simulink model "TosAeb"  (R2021a)
settings: Solver=FixedStepDiscrete  StartTime=0.0  StopTime=inf  FixedStep=0.01  RelTol=1e-3
undefined symbols (not defined in the file or in its folder): ACCModSts, AccSts1, AEB_ObjectMovingOrientation, AEB_ObjectMovingState, CN_BTCActive, CN_CurrentGearR, CN_CurrentGearR_DHT, CN_PTCActive, CN_VDCActive, CN_VSC_EspOff, CN_VSC_FCW_AEB_Error, CN_VSC_FCW_AEB_NoDispay, CN_VSC_FCW_AEB_PerfmDgdtn, CN_VSC_FCW_AEB_TempUnAvlbl, CN_VSC_VehStandstill, CNaccelAvoidMax, CNaccelAvoidMin, CNbrakePedalPress, CNcomfortStrLeftAccelMax, CNcomfortStrLeftAccelMin, CNcomfortStrRightAccelMax, CNcomfortStrRightAccelMin, CNDrvDoorOpen, CNDrvModeEco, CNDrvModeNorm, CNDrvModeOther, CNDrvModeSport, CNDrvSBRreminder, CNNull, CNobjInvalidID, CNPassDoorOpen, CNPassSBRreminder, CNPEBSAccelNegInf, CNPEBSAccelNull, CNPEBSTimeInf, CNPEBSTimeNull, CNSTOPMAX, CNSTOPNULL, CNsubjWidth, CNSwtOFF, CNSwtON, CNTTCMAX, CNTTCNULL, CNVehConfigAEBCycNone, CNVehConfigAEBNone, CNVehConfigAEBPedNone, CNVehConfigFCWNone, CNVehConfigJANone, CollisionSeverity, FALSE, FCWSnvty, JACycObjCalcInfoDefaultParams, JAPedObjCalcInfoDefaultParams, JAVehObjCalcInfoDefaultParams, latCycObjCalcInfoDefaultParams, latPedObjCalcInfoDefaultParams, longObjCalcInfoDefaultParams, ModSts, movingStatus, ObjectType ... (+1112)
libraries: simulink x3031, AEBSFunctionLib x50, Ta_Library x33, AEBSStateMachineLib x23, Mylibrary x12, simulink_extras x7, ObjSelect_lib x3, dspstat3 x2
(part 50 of 102)

== system TosAeb/FcwAebJa_Step_sys/ALG_AEBSystem/ALG_AEBInput/JaObjInfoMap/JaObjInfoMap/Subsystem1/ObjType_Subsystem1/InputObjectType ==
  note: 输出数值为2，类型为car
  note: 输出数值为3，类型为truck
  note: 输出数值为6，类型为pedestrian行人
  note: 输出数值为4，类型为motorbike摩托车
  note: 输出数值为5，类型为bicycle自行车
  note: 输出数值为7，类型为general_object
  note: 输出数值为1，类型为unknown
  InputObjType : Inport
  Compare To Constant : simulink/Logic and Bit Operations/Compare To Constant  relop===  const=0  OutDataTypeStr=boolean
  Compare To Constant1 : simulink/Logic and Bit Operations/Compare To Constant  relop===  const=1  OutDataTypeStr=boolean
  Compare To Constant10 : simulink/Logic and Bit Operations/Compare To Constant  relop===  const=7  OutDataTypeStr=boolean
  Compare To Constant11 : simulink/Logic and Bit Operations/Compare To Constant  relop===  const=9  OutDataTypeStr=boolean
  Compare To Constant12 : simulink/Logic and Bit Operations/Compare To Constant  relop===  const=8  OutDataTypeStr=boolean
  Compare To Constant13 : simulink/Logic and Bit Operations/Compare To Constant  relop===  const=15  OutDataTypeStr=boolean
  Compare To Constant14 : simulink/Logic and Bit Operations/Compare To Constant  relop===  const=10  OutDataTypeStr=boolean
  Compare To Constant15 : simulink/Logic and Bit Operations/Compare To Constant  relop===  const=11  OutDataTypeStr=boolean
  Compare To Constant16 : simulink/Logic and Bit Operations/Compare To Constant  relop===  const=12  OutDataTypeStr=boolean
  Compare To Constant17 : simulink/Logic and Bit Operations/Compare To Constant  relop===  const=14  OutDataTypeStr=boolean
  Compare To Constant18 : simulink/Logic and Bit Operations/Compare To Constant  relop===  const=12  OutDataTypeStr=boolean
  Compare To Constant2 : simulink/Logic and Bit Operations/Compare To Constant  relop===  const=16  OutDataTypeStr=boolean
  Compare To Constant3 : simulink/Logic and Bit Operations/Compare To Constant  relop===  const=2  OutDataTypeStr=boolean
  Compare To Constant4 : simulink/Logic and Bit Operations/Compare To Constant  relop===  const=3  OutDataTypeStr=boolean
  Compare To Constant5 : simulink/Logic and Bit Operations/Compare To Constant  relop===  const=4  OutDataTypeStr=boolean
  Compare To Constant6 : simulink/Logic and Bit Operations/Compare To Constant  relop===  const=5  OutDataTypeStr=boolean
  Compare To Constant7 : simulink/Logic and Bit Operations/Compare To Constant  relop===  const=18  OutDataTypeStr=boolean
  Compare To Constant8 : simulink/Logic and Bit Operations/Compare To Constant  relop===  const=17  OutDataTypeStr=boolean
  Compare To Constant9 : simulink/Logic and Bit Operations/Compare To Constant  relop===  const=6  OutDataTypeStr=boolean
  Logical Operator : Logic  Operator=OR  Inputs=4  AllPortsSameDT=off  OutDataTypeStr=boolean
  Logical Operator1 : Logic  Operator=OR  Inputs=5  AllPortsSameDT=off  OutDataTypeStr=boolean
  Logical Operator2 : Logic  Operator=OR  AllPortsSameDT=off  OutDataTypeStr=boolean
  Logical Operator3 : Logic  Operator=OR  AllPortsSameDT=off  OutDataTypeStr=boolean
  Logical Operator5 : Logic  Operator=OR  Inputs=4  AllPortsSameDT=off  OutDataTypeStr=boolean
  Input_ObjCar : Outport
  Input_ObjTruck : Outport  Port=2
  Input_ObjPed : Outport  Port=3
  Input_ObjMotor : Outport  Port=4
  Input_ObjBicycle : Outport  Port=5
  Input_ObjGeneral : Outport  Port=6
  Input_Unknown : Outport  Port=7
  wires:
  Compare To Constant12/1 -> Logical Operator3/1
  Compare To Constant6/1 -> Logical Operator1/4
  Logical Operator/1 -> Input_ObjCar/1
  Compare To Constant13/1 -> Logical Operator3/2
  InputObjType/1 -> Compare To Constant8/1, Compare To Constant18/1, Compare To Constant17/1, Compare To Constant16/1, Compare To Constant15/1, Compare To Constant14/1, Compare To Constant13/1, Compare To Constant12/1, Compare To Constant11/1, Compare To Constant10/1, Compare To Constant9/1, Compare To Constant7/1, Compare To Constant6/1, Compare To Constant5/1, Compare To Constant4/1, Compare To Constant3/1, Compare To Constant2/1, Compare To Constant1/1, Compare To Constant/1
  Compare To Constant2/1 -> Logical Operator/3
  Compare To Constant1/1 -> Logical Operator/2
  Compare To Constant16/1 -> Logical Operator5/3
  Compare To Constant18/1 -> Input_Unknown/1
  Compare To Constant15/1 -> Logical Operator5/2
  Compare To Constant10/1 -> Logical Operator2/1
  Logical Operator5/1 -> Input_ObjGeneral/1
  Compare To Constant5/1 -> Logical Operator1/3
  Logical Operator3/1 -> Input_ObjBicycle/1
  Logical Operator1/1 -> Input_ObjTruck/1
  Compare To Constant14/1 -> Logical Operator5/1
  Compare To Constant3/1 -> Logical Operator1/1
  Logical Operator2/1 -> Input_ObjMotor/1
  Compare To Constant7/1 -> Logical Operator1/5
  Compare To Constant4/1 -> Logical Operator1/2
  Compare To Constant11/1 -> Logical Operator2/2
  Compare To Constant8/1 -> Logical Operator/4
  Compare To Constant/1 -> Logical Operator/1
  Compare To Constant17/1 -> Logical Operator5/4
  Compare To Constant9/1 -> Input_ObjPed/1

== system TosAeb/FcwAebJa_Step_sys/ALG_AEBSystem/ALG_AEBInput/LatObjInfoMap/latObjInfoMap/Subsystem/MovingStatus_Subsystem/InputMovingStatus ==
  note: 输出数值为3，类型为Moving
  note: 输出数值为2，类型为Stopped
  note: 输出数值为1，类型为Stationary
  note: 输出数值为0，类型为Unknown
  MovingStatus : Inport
  Compare To Constant : simulink/Logic and Bit Operations/Compare To Constant  relop===  const=0  OutDataTypeStr=boolean
  Compare To Constant1 : simulink/Logic and Bit Operations/Compare To Constant  relop===  const=1  OutDataTypeStr=boolean
  Compare To Constant2 : simulink/Logic and Bit Operations/Compare To Constant  relop===  const=3  OutDataTypeStr=boolean
  Compare To Constant8 : simulink/Logic and Bit Operations/Compare To Constant  relop===  const=2  OutDataTypeStr=boolean
  Input_Unknown : Outport
  Input_Stationary : Outport  Port=2
  Input_Stopped : Outport  Port=3
  Input_Moving : Outport  Port=4
  wires:
  Compare To Constant8/1 -> Input_Moving/1
  Compare To Constant1/1 -> Input_Stationary/1
  Compare To Constant2/1 -> Input_Stopped/1
  MovingStatus/1 -> Compare To Constant/1, Compare To Constant1/1, Compare To Constant2/1, Compare To Constant8/1
  Compare To Constant/1 -> Input_Unknown/1

== system TosAeb/FcwAebJa_Step_sys/ALG_AEBSystem/ALG_AEBInput/LatObjInfoMap/latObjInfoMap/Subsystem/ObjType_Subsystem1/InputObjectType ==
  note: 输出数值为2，类型为car
  note: 输出数值为3，类型为truck
  note: 输出数值为6，类型为pedestrian行人
  note: 输出数值为4，类型为motorbike摩托车
  note: 输出数值为5，类型为bicycle自行车
  note: 输出数值为7，类型为general_object
  note: 输出数值为1，类型为unknown
  InputObjType : Inport
  Compare To Constant : simulink/Logic and Bit Operations/Compare To Constant  relop===  const=0  OutDataTypeStr=boolean
  Compare To Constant1 : simulink/Logic and Bit Operations/Compare To Constant  relop===  const=1  OutDataTypeStr=boolean
  Compare To Constant10 : simulink/Logic and Bit Operations/Compare To Constant  relop===  const=7  OutDataTypeStr=boolean
  Compare To Constant11 : simulink/Logic and Bit Operations/Compare To Constant  relop===  const=9  OutDataTypeStr=boolean
  Compare To Constant12 : simulink/Logic and Bit Operations/Compare To Constant  relop===  const=8  OutDataTypeStr=boolean
  Compare To Constant13 : simulink/Logic and Bit Operations/Compare To Constant  relop===  const=15  OutDataTypeStr=boolean
  Compare To Constant14 : simulink/Logic and Bit Operations/Compare To Constant  relop===  const=10  OutDataTypeStr=boolean
  Compare To Constant15 : simulink/Logic and Bit Operations/Compare To Constant  relop===  const=11  OutDataTypeStr=boolean
  Compare To Constant16 : simulink/Logic and Bit Operations/Compare To Constant  relop===  const=12  OutDataTypeStr=boolean
  Compare To Constant17 : simulink/Logic and Bit Operations/Compare To Constant  relop===  const=14  OutDataTypeStr=boolean
  Compare To Constant18 : simulink/Logic and Bit Operations/Compare To Constant  relop===  const=12  OutDataTypeStr=boolean
  Compare To Constant2 : simulink/Logic and Bit Operations/Compare To Constant  relop===  const=16  OutDataTypeStr=boolean
  Compare To Constant3 : simulink/Logic and Bit Operations/Compare To Constant  relop===  const=2  OutDataTypeStr=boolean
  Compare To Constant4 : simulink/Logic and Bit Operations/Compare To Constant  relop===  const=3  OutDataTypeStr=boolean
  Compare To Constant5 : simulink/Logic and Bit Operations/Compare To Constant  relop===  const=4  OutDataTypeStr=boolean
  Compare To Constant6 : simulink/Logic and Bit Operations/Compare To Constant  relop===  const=5  OutDataTypeStr=boolean
  Compare To Constant7 : simulink/Logic and Bit Operations/Compare To Constant  relop===  const=18  OutDataTypeStr=boolean
  Compare To Constant8 : simulink/Logic and Bit Operations/Compare To Constant  relop===  const=17  OutDataTypeStr=boolean
  Compare To Constant9 : simulink/Logic and Bit Operations/Compare To Constant  relop===  const=6  OutDataTypeStr=boolean
  Logical Operator : Logic  Operator=OR  Inputs=4  AllPortsSameDT=off  OutDataTypeStr=boolean
  Logical Operator1 : Logic  Operator=OR  Inputs=5  AllPortsSameDT=off  OutDataTypeStr=boolean
  Logical Operator2 : Logic  Operator=OR  AllPortsSameDT=off  OutDataTypeStr=boolean
  Logical Operator3 : Logic  Operator=OR  AllPortsSameDT=off  OutDataTypeStr=boolean
  Logical Operator5 : Logic  Operator=OR  Inputs=4  AllPortsSameDT=off  OutDataTypeStr=boolean
  Input_ObjCar : Outport
  Input_ObjTruck : Outport  Port=2
  Input_ObjPed : Outport  Port=3
  Input_ObjMotor : Outport  Port=4
  Input_ObjBicycle : Outport  Port=5
  Input_ObjGeneral : Outport  Port=6
  Input_Unknown : Outport  Port=7
  wires:
  Compare To Constant12/1 -> Logical Operator3/1
  Compare To Constant6/1 -> Logical Operator1/4
  Logical Operator/1 -> Input_ObjCar/1
  Compare To Constant13/1 -> Logical Operator3/2
  InputObjType/1 -> Compare To Constant8/1, Compare To Constant18/1, Compare To Constant17/1, Compare To Constant16/1, Compare To Constant15/1, Compare To Constant14/1, Compare To Constant13/1, Compare To Constant12/1, Compare To Constant11/1, Compare To Constant10/1, Compare To Constant9/1, Compare To Constant7/1, Compare To Constant6/1, Compare To Constant5/1, Compare To Constant4/1, Compare To Constant3/1, Compare To Constant2/1, Compare To Constant1/1, Compare To Constant/1
  Compare To Constant2/1 -> Logical Operator/3
  Compare To Constant1/1 -> Logical Operator/2
  Compare To Constant16/1 -> Logical Operator5/3
  Compare To Constant18/1 -> Input_Unknown/1
  Compare To Constant15/1 -> Logical Operator5/2
  Compare To Constant10/1 -> Logical Operator2/1
  Logical Operator5/1 -> Input_ObjGeneral/1
  Compare To Constant5/1 -> Logical Operator1/3
  Logical Operator3/1 -> Input_ObjBicycle/1
  Logical Operator1/1 -> Input_ObjTruck/1
  Compare To Constant14/1 -> Logical Operator5/1
  Compare To Constant3/1 -> Logical Operator1/1
  Logical Operator2/1 -> Input_ObjMotor/1
  Compare To Constant7/1 -> Logical Operator1/5
  Compare To Constant4/1 -> Logical Operator1/2
  Compare To Constant11/1 -> Logical Operator2/2
  Compare To Constant8/1 -> Logical Operator/4
  Compare To Constant/1 -> Logical Operator/1
  Compare To Constant17/1 -> Logical Operator5/4
  Compare To Constant9/1 -> Input_ObjPed/1

== system TosAeb/FcwAebJa_Step_sys/ALG_AEBSystem/ALG_AEBInput/LatObjInfoMap/latObjInfoMap/Subsystem1/MovingStatus_Subsystem/InputMovingStatus ==
  note: 输出数值为3，类型为Moving
  note: 输出数值为2，类型为Stopped
  note: 输出数值为1，类型为Stationary
  note: 输出数值为0，类型为Unknown
  MovingStatus : Inport
  Compare To Constant : simulink/Logic and Bit Operations/Compare To Constant  relop===  const=0  OutDataTypeStr=boolean
  Compare To Constant1 : simulink/Logic and Bit Operations/Compare To Constant  relop===  const=1  OutDataTypeStr=boolean
  Compare To Constant2 : simulink/Logic and Bit Operations/Compare To Constant  relop===  const=3  OutDataTypeStr=boolean
  Compare To Constant8 : simulink/Logic and Bit Operations/Compare To Constant  relop===  const=2  OutDataTypeStr=boolean
  Input_Unknown : Outport
  Input_Stationary : Outport  Port=2
  Input_Stopped : Outport  Port=3
  Input_Moving : Outport  Port=4
  wires:
  Compare To Constant8/1 -> Input_Moving/1
  Compare To Constant1/1 -> Input_Stationary/1
  Compare To Constant2/1 -> Input_Stopped/1
  MovingStatus/1 -> Compare To Constant/1, Compare To Constant1/1, Compare To Constant2/1, Compare To Constant8/1
  Compare To Constant/1 -> Input_Unknown/1

== system TosAeb/FcwAebJa_Step_sys/ALG_AEBSystem/ALG_AEBInput/LatObjInfoMap/latObjInfoMap/Subsystem1/ObjType_Subsystem1/InputObjectType ==
  note: 输出数值为2，类型为car
  note: 输出数值为3，类型为truck
  note: 输出数值为6，类型为pedestrian行人
  note: 输出数值为4，类型为motorbike摩托车
  note: 输出数值为5，类型为bicycle自行车
  note: 输出数值为7，类型为general_object
  note: 输出数值为1，类型为unknown
  InputObjType : Inport
  Compare To Constant : simulink/Logic and Bit Operations/Compare To Constant  relop===  const=0  OutDataTypeStr=boolean
  Compare To Constant1 : simulink/Logic and Bit Operations/Compare To Constant  relop===  const=1  OutDataTypeStr=boolean
  Compare To Constant10 : simulink/Logic and Bit Operations/Compare To Constant  relop===  const=7  OutDataTypeStr=boolean
  Compare To Constant11 : simulink/Logic and Bit Operations/Compare To Constant  relop===  const=9  OutDataTypeStr=boolean
  Compare To Constant12 : simulink/Logic and Bit Operations/Compare To Constant  relop===  const=8  OutDataTypeStr=boolean
  Compare To Constant13 : simulink/Logic and Bit Operations/Compare To Constant  relop===  const=15  OutDataTypeStr=boolean
  Compare To Constant14 : simulink/Logic and Bit Operations/Compare To Constant  relop===  const=10  OutDataTypeStr=boolean
  Compare To Constant15 : simulink/Logic and Bit Operations/Compare To Constant  relop===  const=11  OutDataTypeStr=boolean
  Compare To Constant16 : simulink/Logic and Bit Operations/Compare To Constant  relop===  const=12  OutDataTypeStr=boolean
  Compare To Constant17 : simulink/Logic and Bit Operations/Compare To Constant  relop===  const=14  OutDataTypeStr=boolean
  Compare To Constant18 : simulink/Logic and Bit Operations/Compare To Constant  relop===  const=12  OutDataTypeStr=boolean
  Compare To Constant2 : simulink/Logic and Bit Operations/Compare To Constant  relop===  const=16  OutDataTypeStr=boolean
  Compare To Constant3 : simulink/Logic and Bit Operations/Compare To Constant  relop===  const=2  OutDataTypeStr=boolean
  Compare To Constant4 : simulink/Logic and Bit Operations/Compare To Constant  relop===  const=3  OutDataTypeStr=boolean
  Compare To Constant5 : simulink/Logic and Bit Operations/Compare To Constant  relop===  const=4  OutDataTypeStr=boolean
  Compare To Constant6 : simulink/Logic and Bit Operations/Compare To Constant  relop===  const=5  OutDataTypeStr=boolean
  Compare To Constant7 : simulink/Logic and Bit Operations/Compare To Constant  relop===  const=18  OutDataTypeStr=boolean
  Compare To Constant8 : simulink/Logic and Bit Operations/Compare To Constant  relop===  const=17  OutDataTypeStr=boolean
  Compare To Constant9 : simulink/Logic and Bit Operations/Compare To Constant  relop===  const=6  OutDataTypeStr=boolean
  Logical Operator : Logic  Operator=OR  Inputs=4  AllPortsSameDT=off  OutDataTypeStr=boolean
  Logical Operator1 : Logic  Operator=OR  Inputs=5  AllPortsSameDT=off  OutDataTypeStr=boolean
  Logical Operator2 : Logic  Operator=OR  AllPortsSameDT=off  OutDataTypeStr=boolean
  Logical Operator3 : Logic  Operator=OR  AllPortsSameDT=off  OutDataTypeStr=boolean
  Logical Operator5 : Logic  Operator=OR  Inputs=4  AllPortsSameDT=off  OutDataTypeStr=boolean
  Input_ObjCar : Outport
  Input_ObjTruck : Outport  Port=2
  Input_ObjPed : Outport  Port=3
  Input_ObjMotor : Outport  Port=4
  Input_ObjBicycle : Outport  Port=5
  Input_ObjGeneral : Outport  Port=6
  Input_Unknown : Outport  Port=7
  wires:
  Compare To Constant12/1 -> Logical Operator3/1
  Compare To Constant6/1 -> Logical Operator1/4
  Logical Operator/1 -> Input_ObjCar/1
  Compare To Constant13/1 -> Logical Operator3/2
  InputObjType/1 -> Compare To Constant8/1, Compare To Constant18/1, Compare To Constant17/1, Compare To Constant16/1, Compare To Constant15/1, Compare To Constant14/1, Compare To Constant13/1, Compare To Constant12/1, Compare To Constant11/1, Compare To Constant10/1, Compare To Constant9/1, Compare To Constant7/1, Compare To Constant6/1, Compare To Constant5/1, Compare To Constant4/1, Compare To Constant3/1, Compare To Constant2/1, Compare To Constant1/1, Compare To Constant/1
  Compare To Constant2/1 -> Logical Operator/3
  Compare To Constant1/1 -> Logical Operator/2
  Compare To Constant16/1 -> Logical Operator5/3
  Compare To Constant18/1 -> Input_Unknown/1
  Compare To Constant15/1 -> Logical Operator5/2
  Compare To Constant10/1 -> Logical Operator2/1
  Logical Operator5/1 -> Input_ObjGeneral/1
  Compare To Constant5/1 -> Logical Operator1/3
  Logical Operator3/1 -> Input_ObjBicycle/1
  Logical Operator1/1 -> Input_ObjTruck/1
  Compare To Constant14/1 -> Logical Operator5/1
  Compare To Constant3/1 -> Logical Operator1/1
  Logical Operator2/1 -> Input_ObjMotor/1
  Compare To Constant7/1 -> Logical Operator1/5
  Compare To Constant4/1 -> Logical Operator1/2
  Compare To Constant11/1 -> Logical Operator2/2
  Compare To Constant8/1 -> Logical Operator/4
  Compare To Constant/1 -> Logical Operator/1
  Compare To Constant17/1 -> Logical Operator5/4
  Compare To Constant9/1 -> Input_ObjPed/1

== system TosAeb/AEB_Objsel/TA_Processor/AEB_ObjSelect/TargetInfoCheck/Data_precess/TA_EffectCalculate/Obj_FilterFlgCalculate/JA_ObjFilter/CollisionProbCalc ==
  tPredTime : Inport  OutDataTypeStr=single
  subjVel : Inport  Port=2  OutDataTypeStr=single
  subjLongAccel : Inport  Port=3  OutDataTypeStr=single
  subjLatAccel : Inport  Port=4  OutDataTypeStr=single
  subjYawRate : Inport  Port=5  OutDataTypeStr=single
  ObjectYawAngle : Inport  Port=6  OutDataTypeStr=single
  ObjectMovingOrientation : Inport  Port=7
  ObjectLength : Inport  Port=8  OutDataTypeStr=single
  ObjectWidth : Inport  Port=9  OutDataTypeStr=single
  dx : Inport  Port=10  OutDataTypeStr=single
  dy : Inport  Port=11  OutDataTypeStr=single
  ay : Inport  Port=12  OutDataTypeStr=single
  ObjectID : Inport  Port=13
  vy : Inport  Port=14  OutDataTypeStr=single
  vxAbsObj : Inport  Port=15  OutDataTypeStr=single
  axAbsObj : Inport  Port=16  OutDataTypeStr=single
  PahEstimn : Inport  Port=17
  function : TriggerPort  TriggerType=function-call  VariantControl=Variant  PortDimensions=1
  If : If  IfExpression=u1
  If Action Subsystem : Subsystem  TreatAsAtomicUnit=on
  Merge : Merge
  ObjIsInvalidCalc : Subsystem
  Subsystem : Subsystem
  Terminator : Terminator
  ObjColliFlg : Outport
  wires:
  tPredTime/1 -> Subsystem/1(tPredTime)
  subjVel/1 -> Subsystem/2(subjVel)
  subjLongAccel/1 -> Subsystem/3(subjLongAccel)
  subjLatAccel/1 -> Subsystem/4(subjLatAccel)
  subjYawRate/1 -> Subsystem/5(subjYawRate)
  ObjectYawAngle/1 -> Subsystem/6(ObjectYawAngle)
  ObjectLength/1 -> Subsystem/7(ObjectLength)
  ObjectWidth/1 -> Subsystem/8(ObjectWidth)
  dx/1 -> Subsystem/9(dx)
  ay/1 -> Subsystem/11(ay)
  vxAbsObj/1 -> Subsystem/13(vxAbsObj)
  axAbsObj/1 -> Subsystem/14(axAbsObj)
  If/1 -> If Action Subsystem/Action
  If Action Subsystem/1(ObjColliFlg) -> Merge/1
  If/2 -> Subsystem/Action
  Merge/1 -> ObjColliFlg/1
  Subsystem/1(ObjColliFlg) -> Merge/2
  dy/1 -> Subsystem/10(dy)
  ObjectID/1 -> ObjIsInvalidCalc/1(ObjectID)
  vy/1 -> Subsystem/12(vy)
  ObjIsInvalidCalc/1(ObjIsInvalid) -> If/1
  PahEstimn/1 -> Subsystem/15(PahEstimn)
  ObjectMovingOrientation/1 -> Terminator/1

== system TosAeb/AEB_Objsel/TA_Processor/AEB_ObjSelect/TargetInfoCheck/Data_precess/TA_EffectCalculate/Obj_FilterFlgCalculate/JA_ObjFilter/JAObjEnable ==
  TA_ObjInfo : Inport
  Bus Selector : BusSelector  OutputSignals=TA_ObsType,TA_ObsVxAbs,TA_ObsConfidence
  JAObjEnableJudge : Subsystem
  JAObjEnable : Outport
  wires:
  TA_ObjInfo/1 -> Bus Selector/1
  Bus Selector/1 -> JAObjEnableJudge/1(TA_ObsType)  "<TA_ObsType>"
  Bus Selector/2 -> JAObjEnableJudge/2(TA_ObsVxAbs)  "<TA_ObsVxAbs>"
  Bus Selector/3 -> JAObjEnableJudge/3(TA_ObsConfidence)  "<TA_ObsConfidence>"
  JAObjEnableJudge/1(JAObjEnable) -> JAObjEnable/1

== system TosAeb/AEB_Objsel/TA_Processor/AEB_ObjSelect/TargetInfoCheck/Data_precess/TA_EffectCalculate/Obj_FilterFlgCalculate/Lat_ObjFilter/Lat_Objelse ==
  TA_ObjInfo : Inport
  VehSelfData : Inport  Port=2
  Action Port : ActionPort  ActionPortLabel=else
  Bus Selector : BusSelector  OutputSignals=TA_ObsType,TA_ObsOrientation,TA_ObsState,TA_ObsVx,TA_ObsVy,TA_ObsDx,TA_ObsDy,TA_ObsAx,TA_ObsAy,TA_ObsWidth,TA_ObsVyAbs
  Bus Selector1 : BusSelector  OutputSignals=VehSelf.ABS_VehicleSpeed,VehSelf.YawRate
  Lat_ObjPre : Subsystem
  LatObj_Valid : Outport
  Lat_ObjIntersect : Outport  Port=2
  Lat_TTR : Outport  Port=3
  wires:
  TA_ObjInfo/1 -> Bus Selector/1
  VehSelfData/1 -> Bus Selector1/1
  Bus Selector1/2 -> Lat_ObjPre/13(YawRate)  "<YawRate>"
  Bus Selector1/1 -> Lat_ObjPre/12(Subj_Vx)  "<ABS_VehicleSpeed>"
  Lat_ObjPre/1(LatObj_Valid) -> LatObj_Valid/1
  Lat_ObjPre/3(Lat_TTR) -> Lat_TTR/1
  Lat_ObjPre/2(Lat_ObjIntersect) -> Lat_ObjIntersect/1
  Bus Selector/1 -> Lat_ObjPre/1(Obj_Type)  "<TA_ObsType>"
  Bus Selector/2 -> Lat_ObjPre/2(Obj_Oreintation)  "<TA_ObsOrientation>"
  Bus Selector/3 -> Lat_ObjPre/3(Obj_State)  "<TA_ObsState>"
  Bus Selector/4 -> Lat_ObjPre/4(Obj_Vx)  "<TA_ObsVx>"
  Bus Selector/5 -> Lat_ObjPre/5(Obj_Vy)  "<TA_ObsVy>"
  Bus Selector/6 -> Lat_ObjPre/6(dx)  "<TA_ObsDx>"
  Bus Selector/7 -> Lat_ObjPre/7(dy)  "<TA_ObsDy>"
  Bus Selector/8 -> Lat_ObjPre/8(ax)  "<TA_ObsAx>"
  Bus Selector/9 -> Lat_ObjPre/9(ay)  "<TA_ObsAy>"
  Bus Selector/10 -> Lat_ObjPre/10(Width)  "<TA_ObsWidth>"
  Bus Selector/11 -> Lat_ObjPre/11(ObsVyAbs)  "<TA_ObsVyAbs>"

== system TosAeb/AEB_Objsel/TA_Processor/AEB_ObjSelect/TargetInfoCheck/Data_precess/TA_EffectCalculate/Obj_FilterFlgCalculate/Lat_ObjFilter/Lat_Objif ==
  Action Port : ActionPort  ActionPortLabel=if(u1 > 0)
  Constant : Constant  Value=0  OutDataTypeStr=boolean
  Constant1 : Constant  Value=0  OutDataTypeStr=boolean
  Constant2 : Constant  Value=100  OutDataTypeStr=single
  LatObj_Valid : Outport
  Lat_ObjIntersect : Outport  Port=2
  Lat_TTR : Outport  Port=3
  wires:
  Constant/1 -> LatObj_Valid/1
  Constant1/1 -> Lat_ObjIntersect/1
  Constant2/1 -> Lat_TTR/1

== system TosAeb/AEB_Objsel/TA_Processor/AEB_ObjSelect/TargetInfoCheck/Data_precess/TA_EffectCalculate/Obj_FilterFlgCalculate/Lat_ObjFilter/Subsystem ==
  TA_ObjID : Inport
  Compare To Constant : simulink/Logic and Bit Operations/Compare To Constant  relop===  const=0  OutDataTypeStr=boolean
  Data Type Conversion : DataTypeConversion
  Obj_IDFlg : Outport
  wires:
  TA_ObjID/1 -> Compare To Constant/1
  Compare To Constant/1 -> Data Type Conversion/1
  Data Type Conversion/1 -> Obj_IDFlg/1

== system TosAeb/AEB_Objsel/TA_Processor/AEB_ObjSelect/TargetInfoCheck/Data_precess/TA_EffectCalculate/Obj_FilterFlgCalculate/Long_ObjFilter/Info_Calculate ==
  Obj_WeightCoef : Inport
  TA_ObjInfo : Inport  Port=2
  VehSelfData : Inport  Port=3
  Bus Selector : BusSelector  OutputSignals=TA_ObsOrientation,TA_ObsAy,TA_ObsAx,TA_ObsVyStd,TA_ObsVy,TA_ObsVxStd,TA_ObsVx,TA_ObsState,TA_ObsHeadAngle
  Bus Selector1 : BusSelector  OutputSignals=VehSelf.ABS_VehicleSpeed,VehSelf.YawRate,VehSelf.AY1,VehSelf.ABS_MasterCylinderPressure,VehSelf.AEB_Intervention_ESP
  If : If
  Merge : Merge
  ObjWeight_Invalid : Subsystem  TreatAsAtomicUnit=on
  ObjWeight_Jugement : Subsystem
  Subsystem : Subsystem
  Long_ObjWeightCoef : Outport
  wires:
  Obj_WeightCoef/1 -> Subsystem/1(Obj_WeightCoef), ObjWeight_Jugement/1(Obj_WeightCoef)
  Subsystem/1(Obj_Coeflg) -> If/1
  If/1 -> ObjWeight_Invalid/Action
  If/2 -> ObjWeight_Jugement/Action
  ObjWeight_Invalid/1(Long_ObjWeightCoef) -> Merge/1
  ObjWeight_Jugement/1(Long_ObjWeightCoef) -> Merge/2
  Merge/1 -> Long_ObjWeightCoef/1
  TA_ObjInfo/1 -> Bus Selector/1
  VehSelfData/1 -> Bus Selector1/1
  Bus Selector1/1 -> ObjWeight_Jugement/11(Subj_Vx)  "<ABS_VehicleSpeed>"
  Bus Selector1/2 -> ObjWeight_Jugement/12(Subj_Yaw)  "<YawRate>"
  Bus Selector1/3 -> ObjWeight_Jugement/13(Subj_Ay)  "<AY1>"
  Bus Selector1/4 -> ObjWeight_Jugement/14(Subj_Decel)  "<ABS_MasterCylinderPressure>"
  Bus Selector/1 -> ObjWeight_Jugement/2(Obj_Oreintation)  "<TA_ObsOrientation>"
  Bus Selector/2 -> ObjWeight_Jugement/3(Obj_Accy)  "<TA_ObsAy>"
  Bus Selector/3 -> ObjWeight_Jugement/4(Obj_Accx)  "<TA_ObsAx>"
  Bus Selector/4 -> ObjWeight_Jugement/5(Obj_VyStd)  "<TA_ObsVyStd>"
  Bus Selector/5 -> ObjWeight_Jugement/6(Obj_Vy)  "<TA_ObsVy>"
  Bus Selector/6 -> ObjWeight_Jugement/7(Obj_VxStd)  "<TA_ObsVxStd>"
  Bus Selector/7 -> ObjWeight_Jugement/8(Obj_Vx)  "<TA_ObsVx>"
  Bus Selector/8 -> ObjWeight_Jugement/9(Obj_State)  "<TA_ObsState>"
  Bus Selector1/5 -> ObjWeight_Jugement/15(AEB_State)  "<AEB_Intervention_ESP>"
  Bus Selector/9 -> ObjWeight_Jugement/10(Obj_HeadAg)  "<TA_ObsHeadAngle>"

== system TosAeb/AEB_Objsel/TA_Processor/AEB_ObjSelect/TargetInfoCheck/Data_precess/TA_EffectCalculate/Obj_FilterFlgCalculate/Long_ObjFilter/OverlapCalculate ==
  Long_ObjWeightCoefTTI : Inport
  TimeToReachLgt : Inport  Port=2
  PahEstimn : Inport  Port=3
  TA_ObjInfo : Inport  Port=4
  VehSelfData : Inport  Port=5
  BoudingBoxCalculate_Else : Subsystem
  BoudingBoxCalculate_if : Subsystem  TreatAsAtomicUnit=on
  If : If
  Merge : Merge
  Subsystem : Subsystem
  Boudingbox_Calculate : Outport
  wires:
  Long_ObjWeightCoefTTI/1 -> Subsystem/1(Obj_WeightCoef)
  Subsystem/1(Obj_Coeflg) -> If/1
  If/1 -> BoudingBoxCalculate_if/Action
  TimeToReachLgt/1 -> BoudingBoxCalculate_Else/1(TimeToReachLgt)
  PahEstimn/1 -> BoudingBoxCalculate_Else/2(PahEstimn)  "PahEstimn"
  TA_ObjInfo/1 -> BoudingBoxCalculate_Else/3(TA_ObjInfo)
  VehSelfData/1 -> BoudingBoxCalculate_Else/4(VehSelfData)
  If/2 -> BoudingBoxCalculate_Else/Action
  BoudingBoxCalculate_if/1(Boudingbox_Calculate) -> Merge/1
  BoudingBoxCalculate_Else/1(Boudingbox_Calculate) -> Merge/2
  Merge/1 -> Boudingbox_Calculate/1

== system TosAeb/AEB_Objsel/TA_Processor/AEB_ObjSelect/TargetInfoCheck/Data_precess/TA_EffectCalculate/Obj_FilterFlgCalculate/Long_ObjFilter/TTR_Calculate ==
  Long_ObjWeightCoef : Inport
  TA_ObjInfo : Inport  Port=2
  VehSelfData : Inport  Port=3
  CalculateTTRAndTTM_else : Subsystem
  CalculateTTRAndTTM_if : Subsystem  TreatAsAtomicUnit=on
  If : If
  Merge : Merge
  Merge1 : Merge
  Subsystem : Subsystem
  Long_ObjWeightCoefTTI : Outport
  TimeToReachLgt : Outport  Port=2
  wires:
  Long_ObjWeightCoef/1 -> CalculateTTRAndTTM_else/1(Long_ObjWeightCoef), Subsystem/1(Obj_WeightCoef)
  TA_ObjInfo/1 -> CalculateTTRAndTTM_else/2(TA_ObjInfo)
  VehSelfData/1 -> CalculateTTRAndTTM_else/3(VehSelfData)
  If/1 -> CalculateTTRAndTTM_if/Action
  Subsystem/1(Obj_Coeflg) -> If/1
  CalculateTTRAndTTM_else/1(Long_ObjWeightCoefTTI) -> Merge/2
  CalculateTTRAndTTM_if/1(Long_ObjWeightCoefTTI) -> Merge/1
  Merge/1 -> Long_ObjWeightCoefTTI/1
  If/2 -> CalculateTTRAndTTM_else/Action
  CalculateTTRAndTTM_if/2(TimeToReachLgt) -> Merge1/1
  CalculateTTRAndTTM_else/2(TimeToReachLgt) -> Merge1/2
  Merge1/1 -> TimeToReachLgt/1

== system TosAeb/AEB_Objsel/TA_Processor/AEB_ObjSelect/TargetInfoCheck/Data_precess/TA_EffectCalculate/ValideateObj/ObjPos_Calculate/ObjPos_Calculateelse ==
  TA_ObsInfo : Inport
  PathEstimn : Inport  Port=2
  Action Port : ActionPort  ActionPortLabel=else
  Bus Selector : BusSelector  OutputSignals=TA_ObsLength,TA_ObsWidth,TA_ObsDx,TA_ObsDy,TA_ObsVy,TA_ObsHeadAngle,TA_ObsAyAbs,TA_ObsVyAbs,TA_ObsVx
  InPath_Cal : Subsystem
  Unit Delay : UnitDelay  SampleTime=-1  HasFrameUpgradeWarning=on
  Overlap_Current : Outport
  Overlap_Prediction : Outport  Port=2
  wires:
  PathEstimn/1 -> InPath_Cal/10(PathEstimn)
  TA_ObsInfo/1 -> Bus Selector/1
  Bus Selector/3 -> InPath_Cal/3(TA_ObjDx)  "<TA_ObsDx>"
  Bus Selector/2 -> InPath_Cal/2(TA_ObjWidth)  "<TA_ObsWidth>"
  Bus Selector/4 -> InPath_Cal/4(TA_ObjDy)  "<TA_ObsDy>"
  Bus Selector/1 -> InPath_Cal/1(TA_ObjLength)  "<TA_ObsLength>"
  Bus Selector/5 -> InPath_Cal/5(TA_Objvy)  "<TA_ObsVy>"
  Bus Selector/6 -> InPath_Cal/6(TA_HeadingAnlge)  "<TA_ObsHeadAngle>"
  Bus Selector/7 -> InPath_Cal/7(Obj_AbsAccly)  "<TA_ObsAyAbs>"
  Bus Selector/8 -> InPath_Cal/8(Obj_AbsSpdy)  "<TA_ObsVyAbs>"
  Bus Selector/9 -> InPath_Cal/9(TA_ObjVx)  "<TA_ObsVx>"
  InPath_Cal/1(Overlap_Current) -> Overlap_Current/1
  InPath_Cal/2(Overlap_Prediction) -> Overlap_Prediction/1
  InPath_Cal/3(Overlap_Rate) -> Unit Delay/1
  Unit Delay/1 -> InPath_Cal/11(Overlap_Ratedelay)

== system TosAeb/AEB_Objsel/TA_Processor/AEB_ObjSelect/TargetInfoCheck/Data_precess/TA_EffectCalculate/ValideateObj/ObjPos_Calculate/ObjPos_Calculateif ==
  Action Port : ActionPort  ActionPortLabel=if(u1 > 0)
  Constant : Constant  Value=0  OutDataTypeStr=boolean
  Constant1 : Constant  Value=0  OutDataTypeStr=boolean
  Overlap_Current : Outport
  Overlap_Prediction : Outport  Port=2
  wires:
  Constant/1 -> Overlap_Current/1
  Constant1/1 -> Overlap_Prediction/1

== system TosAeb/AEB_Objsel/TA_Processor/AEB_ObjSelect/TargetInfoCheck/Data_precess/TA_EffectCalculate/ValideateObj/ObjPos_Calculate/Subsystem ==
  Obj_Enable : Inport
  Compare To Constant : simulink/Logic and Bit Operations/Compare To Constant  relop===  const=0  OutDataTypeStr=boolean
  Compare To Constant1 : simulink/Logic and Bit Operations/Compare To Constant  relop===  const=1  OutDataTypeStr=boolean
  Data Type Conversion : DataTypeConversion
  Utility : Outport
  Obj_Flg : Outport  Port=2
  wires:
  Obj_Enable/1 -> Compare To Constant/1, Compare To Constant1/1
  Compare To Constant/1 -> Data Type Conversion/1
  Data Type Conversion/1 -> Obj_Flg/1
  Compare To Constant1/1 -> Utility/1

== system TosAeb/AEB_Objsel/TA_Processor/AEB_ObjSelect/TargetInfoCheck/Data_precess/TA_EffectCalculate/ValideateObj/ValidObj_PreProcess/If Action Subsystem ==
  Action Port : ActionPort  ActionPortLabel=if(u1 > 0)
  Constant : Constant  Value=0  OutDataTypeStr=boolean
  Obj_Enable : Outport
  wires:
  Constant/1 -> Obj_Enable/1

== system TosAeb/AEB_Objsel/TA_Processor/AEB_ObjSelect/TargetInfoCheck/Data_precess/TA_EffectCalculate/ValideateObj/ValidObj_PreProcess/If Action Subsystem1 ==
  TA_ObsType : Inport
  TA_ObjBehavior : Inport  Port=2
  TA_Obsdx : Inport  Port=3
  TA_Obsdy : Inport  Port=4
  TA_Obsvx : Inport  Port=5
  TA_ObsQuality : Inport  Port=6
  TA_ObsVxAbs : Inport  Port=7
  Veh_LongSpd_mps : Inport  Port=8
  Action Port : ActionPort  ActionPortLabel=else
  CheckBehavior : Subsystem
  CheckPosition : Subsystem
  CheckQuality : Subsystem
  CheckType : Subsystem
  Logical Operator : Logic  Inputs=4  AllPortsSameDT=off  OutDataTypeStr=boolean
  Obj_Enable : Outport
  wires:
  TA_ObsType/1 -> CheckType/1(TA_ObsType)
  TA_ObjBehavior/1 -> CheckBehavior/1(TA_ObjBehavior)
  TA_Obsdx/1 -> CheckPosition/1(TA_Obsdx)
  TA_Obsdy/1 -> CheckPosition/2(TA_Obsdy)
  TA_Obsvx/1 -> CheckBehavior/2(TA_Objvx)
  Veh_LongSpd_mps/1 -> CheckBehavior/3(Veh_LongSpd_mps)
  TA_ObsQuality/1 -> CheckQuality/1(TA_ObsQuality)
  CheckBehavior/1(Obj_BehaviorEnable) -> Logical Operator/4
  CheckQuality/1(Obj_QualityEnable) -> Logical Operator/3
  CheckPosition/1(Obj_PosEnable) -> Logical Operator/2
  CheckType/1(Obj_TpyeEnable) -> Logical Operator/1
  Logical Operator/1 -> Obj_Enable/1
  TA_ObsVxAbs/1 -> CheckBehavior/4(TA_ObsVxAbs)

== system TosAeb/AEB_Objsel/TA_Processor/AEB_ObjSelect/TargetInfoCheck/Data_precess/TA_EffectCalculate/ValideateObj/ValidObj_PreProcess/Subsystem ==
  TA_ObsID : Inport
  Compare To Constant : simulink/Logic and Bit Operations/Compare To Constant  relop===  const=0  OutDataTypeStr=boolean
  Data Type Conversion : DataTypeConversion
  Obj_IDFlg : Outport
  wires:
  TA_ObsID/1 -> Compare To Constant/1
  Compare To Constant/1 -> Data Type Conversion/1
  Data Type Conversion/1 -> Obj_IDFlg/1

== system TosAeb/AEB_Objsel/TA_Processor/PreProcessor/ObjectPrecessor/TAObjInfoCalc/For Each Subsystem/Info_Check1/BasicInfo_check/AbsSpd ==
  ObsVelXAbs_s : Inport
  ObsVelYAbs_s : Inport  Port=2
  Vhe_Spd : Inport  Port=3
  Veh_YawRate : Inport  Port=4
  Obj_VxCr : Inport  Port=5
  Obj_VyCr : Inport  Port=6
  AbsVx_correct : Subsystem
  AbsVy_correct : Subsystem
  Fus_ObjVxAbs : Outport
  Fus_ObjVyAbs : Outport  Port=2
  wires:
  ObsVelXAbs_s/1 -> AbsVx_correct/1(ObsVelXAbs_s)
  Vhe_Spd/1 -> AbsVx_correct/2(Vhe_Spd)
  Obj_VxCr/1 -> AbsVx_correct/3(Obj_VxCr)
  ObsVelYAbs_s/1 -> AbsVy_correct/1(ObsVelYAbs_s)
  Veh_YawRate/1 -> AbsVy_correct/2(Veh_YawRate)
  Obj_VyCr/1 -> AbsVy_correct/3(Obj_VyCr)
  AbsVx_correct/1(Fus_ObjVxAbs) -> Fus_ObjVxAbs/1
  AbsVy_correct/1(Fus_ObjVyAbs) -> Fus_ObjVyAbs/1

== system TosAeb/AEB_Objsel/TA_Processor/PreProcessor/ObjectPrecessor/TAObjInfoCalc/For Each Subsystem/Info_Check1/BasicInfo_check/AccCorrect ==
  ObsAccX_s : Inport
  ObsAccY_s : Inport  Port=2
  Veh_AccX : Inport  Port=3
  Veh_AccY : Inport  Port=4
  Obj_vy : Inport  Port=5
  Obj_Vx : Inport  Port=6
  Veh_AEBSta : Inport  Port=7
  Acc_XY : Subsystem
  Fus_ObjAy : Outport
  Fus_ObjAx : Outport  Port=2
  wires:
  Obj_Vx/1 -> Acc_XY/4(Obj_Vx)
  Obj_vy/1 -> Acc_XY/2(Obj_vy)
  ObsAccY_s/1 -> Acc_XY/1(ObsAccY_s)
  ObsAccX_s/1 -> Acc_XY/3(ObsAccX_s)
  Veh_AccX/1 -> Acc_XY/5(Veh_AccX)
  Veh_AccY/1 -> Acc_XY/6(Veh_AccY)
  Veh_AEBSta/1 -> Acc_XY/7(Veh_AEBSta)
  Acc_XY/1(Fus_ObjAy) -> Fus_ObjAy/1
  Acc_XY/2(Fus_ObjAx) -> Fus_ObjAx/1

== system TosAeb/AEB_Objsel/TA_Processor/PreProcessor/ObjectPrecessor/TAObjInfoCalc/For Each Subsystem/Info_Check1/BasicInfo_check/DxCorrect ==
  Obj_ID : Inport
  Fus_ObjDx_Single : Inport  Port=2
  Fus_ObjDxQua_Single : Inport  Port=3
  Fus_ObjVx_Single : Inport  Port=4
  Veh_AEBFunState : Inport  Port=5
  If : If
  Merge : Merge
  Merge3 : Merge
  Subsystem : Subsystem
  dx_charge : Subsystem
  dx_nocharge : Subsystem  TreatAsAtomicUnit=on
  Fus_ObjDx : Outport
  Dx_CorrectFlg : Outport  Port=2
  wires:
  Merge3/1 -> Dx_CorrectFlg/1
  dx_charge/2(Dx_CorrectFlg) -> Merge3/2
  dx_nocharge/2(Dx_CorrectFlg) -> Merge3/1
  dx_charge/1(Fus_ObjDx) -> Merge/2
  Merge/1 -> Fus_ObjDx/1
  dx_nocharge/1(Fus_ObjDx) -> Merge/1
  If/2 -> dx_charge/Action
  If/1 -> dx_nocharge/Action
  Veh_AEBFunState/1 -> dx_charge/4(Veh_AEBFunState)
  Fus_ObjVx_Single/1 -> dx_charge/3(Fus_ObjVx_Single)
  Fus_ObjDxQua_Single/1 -> dx_charge/2(Fus_ObjDxStd)
  Fus_ObjDx_Single/1 -> dx_nocharge/1(Fus_ObjDx_Single), dx_charge/1(Fus_ObjDx_Single)
  Obj_ID/1 -> Subsystem/1(Obj_ID)
  Subsystem/1(Obj_Invalid) -> If/1

== system TosAeb/AEB_Objsel/TA_Processor/PreProcessor/ObjectPrecessor/TAObjInfoCalc/For Each Subsystem/Info_Check1/BasicInfo_check/DyCorrect ==
  Obj_ID : Inport
  Fus_ObjDy_Single : Inport  Port=2
  Fus_ObsDyStd : Inport  Port=3
  Veh_AEBFunState : Inport  Port=4
  Fus_ObjVy_Single : Inport  Port=5
  If : If
  Merge : Merge
  Merge3 : Merge
  Subsystem : Subsystem
  dy_charge : Subsystem
  dy_nocharge : Subsystem  TreatAsAtomicUnit=on
  Fus_ObjDy : Outport
  Dy_CorrectFlg : Outport  Port=2
  wires:
  Merge3/1 -> Dy_CorrectFlg/1
  dy_charge/2(Dy_CorrectFlg) -> Merge3/2
  dy_nocharge/2(Dy_CorrectFlg) -> Merge3/1
  dy_charge/1(Fus_ObjDy) -> Merge/2
  Merge/1 -> Fus_ObjDy/1
  dy_nocharge/1(Fus_ObjDy) -> Merge/1
  Veh_AEBFunState/1 -> dy_charge/4(Veh_AEBFunState)
  Fus_ObjVy_Single/1 -> dy_charge/3(Fus_ObjVy_Single)
  Fus_ObsDyStd/1 -> dy_charge/2(Fus_ObsDyStd)
  Fus_ObjDy_Single/1 -> dy_charge/1(Fus_ObjDy_Single), dy_nocharge/1(Fus_ObjDy_Single)
  If/2 -> dy_charge/Action
  If/1 -> dy_nocharge/Action
  Subsystem/1(Obj_Invalid) -> If/1
  Obj_ID/1 -> Subsystem/1(Obj_ID)

== system TosAeb/AEB_Objsel/TA_Processor/PreProcessor/ObjectPrecessor/TAObjInfoCalc/For Each Subsystem/Info_Check1/BasicInfo_check/HeadAngle ==
  Obj_ID : Inport
  Fus_Type : Inport  Port=2
  ObsAngle_s : Inport  Port=3
  ObsAngleSTD_s : Inport  Port=4
  Obj_VxCr : Inport  Port=5
  Obj_VyCr : Inport  Port=6
  If Action Subsystem : Subsystem  TreatAsAtomicUnit=on
  If Action Subsystem1 : Subsystem  TreatAsAtomicUnit=on
  If1 : If
  Merge : Merge
  Subsystem : Subsystem
  Fus_ObjHeadAngle : Outport
  wires:
  Subsystem/1(Obj_Invalid) -> If1/1
  Obj_ID/1 -> Subsystem/1(Obj_ID)
  If1/1 -> If Action Subsystem/Action
  If1/2 -> If Action Subsystem1/Action
  Obj_VxCr/1 -> If Action Subsystem1/4(Obj_VxCr)
  Obj_VyCr/1 -> If Action Subsystem1/5(Obj_VyCr)
  ObsAngleSTD_s/1 -> If Action Subsystem1/3(ObsAngleSTD_s)
  ObsAngle_s/1 -> If Action Subsystem1/2(ObsAngle_s)
  Fus_Type/1 -> If Action Subsystem1/1(Fus_Type)
  If Action Subsystem/1(Fus_ObjHeadAngle) -> Merge/1
  If Action Subsystem1/1(Fus_ObjHeadAngle) -> Merge/2
  Merge/1 -> Fus_ObjHeadAngle/1

== system TosAeb/AEB_Objsel/TA_Processor/PreProcessor/ObjectPrecessor/TAObjInfoCalc/For Each Subsystem/Info_Check1/BasicInfo_check/IDProcess ==
  ID_change : Inport
  ObsID_u8 : Inport  Port=2
  Compare To Constant : simulink/Logic and Bit Operations/Compare To Constant  relop===  const=1  OutDataTypeStr=boolean
  Compare To Constant1 : simulink/Logic and Bit Operations/Compare To Constant  relop=~=  const=0  OutDataTypeStr=boolean
  Constant : Constant  OutDataTypeStr=uint8
  Constant1 : Constant  Value=0  OutDataTypeStr=uint8
  Logical Operator : Logic  Operator=OR  AllPortsSameDT=off  OutDataTypeStr=boolean
  Switch : Switch  Criteria=u2 > Threshold
  Obs_ID : Outport
  wires:
  ID_change/1 -> Compare To Constant/1
  ObsID_u8/1 -> Compare To Constant1/1
  Compare To Constant/1 -> Logical Operator/1
  Compare To Constant1/1 -> Logical Operator/2
  Logical Operator/1 -> Switch/2
  Switch/1 -> Obs_ID/1
  Constant/1 -> Switch/1
  Constant1/1 -> Switch/3

== system TosAeb/AEB_Objsel/TA_Processor/PreProcessor/ObjectPrecessor/TAObjInfoCalc/For Each Subsystem/Info_Check1/BasicInfo_check/QuaCorrect ==
  Obj_ID : Inport
  ObsConfidence_u8 : Inport  Port=2
  Veh_AEBFunState : Inport  Port=3
  dy_flg : Inport  Port=4
  dx_flg : Inport  Port=5
  vx_flg : Inport  Port=6
  vy_flg : Inport  Port=7
  If1 : If
  Merge : Merge
  Subsystem : Subsystem
  quality_recrectionelse : Subsystem
  quality_recrectionif : Subsystem  TreatAsAtomicUnit=on
  Fus_ObjQuality : Outport
  wires:
  Merge/1 -> Fus_ObjQuality/1
  quality_recrectionelse/1(Fus_ObjQuality) -> Merge/2
  quality_recrectionif/1(Fus_ObjQuality) -> Merge/1
  If1/1 -> quality_recrectionif/Action
  If1/2 -> quality_recrectionelse/Action
  vy_flg/1 -> quality_recrectionelse/6(vy_flg)
  vx_flg/1 -> quality_recrectionelse/5(vx_flg)
  dx_flg/1 -> quality_recrectionelse/4(dx_flg)
  dy_flg/1 -> quality_recrectionelse/3(dy_flg)
  Veh_AEBFunState/1 -> quality_recrectionelse/2(Veh_AEBFunState)
  ObsConfidence_u8/1 -> quality_recrectionif/1(Fus_TargetQuality), quality_recrectionelse/1(Fus_TargetQuality)
  Subsystem/1(Obj_Invalid) -> If1/1
  Obj_ID/1 -> Subsystem/1(Obj_ID)

== system TosAeb/AEB_Objsel/TA_Processor/PreProcessor/ObjectPrecessor/TAObjInfoCalc/For Each Subsystem/Info_Check1/BasicInfo_check/VxCorrect ==
  Obj_ID : Inport
  Fus_ObjVx_Single : Inport  Port=2
  Fus_ObjVxStd : Inport  Port=3
  Fus_ObjDx_Single : Inport  Port=4
  Veh_AEBFunState : Inport  Port=5
  If : If
  If Action Subsystem : Subsystem  TreatAsAtomicUnit=on
  Merge : Merge
  Merge3 : Merge
  Subsystem : Subsystem
  Subsystem3 : Subsystem
  Fus_ObjVx : Outport
  Vx_CorrectFlg : Outport  Port=2
  wires:
  Merge3/1 -> Vx_CorrectFlg/1
  Subsystem3/2(Vx_CorrectFlg) -> Merge3/2
  If Action Subsystem/2(Vx_CorrectFlg) -> Merge3/1
  Merge/1 -> Fus_ObjVx/1
  Subsystem3/1(Fus_ObjVx) -> Merge/2
  If Action Subsystem/1(Fus_ObjVx) -> Merge/1
  If/2 -> Subsystem3/Action
  Veh_AEBFunState/1 -> Subsystem3/4(Veh_AEBFunState)
  Fus_ObjVxStd/1 -> Subsystem3/3(Fus_ObjVxStd)
  Fus_ObjDx_Single/1 -> Subsystem3/2(Fus_ObjDx_Single)
  Fus_ObjVx_Single/1 -> Subsystem3/1(Fus_ObjVx_Single), If Action Subsystem/1(Fus_ObjVx_Single)
  If/1 -> If Action Subsystem/Action
  Obj_ID/1 -> Subsystem/1(Obj_ID)
  Subsystem/1(Obj_Invalid) -> If/1

== system TosAeb/AEB_Objsel/TA_Processor/PreProcessor/ObjectPrecessor/TAObjInfoCalc/For Each Subsystem/Info_Check1/BasicInfo_check/VyCorrect ==
  Obj_ID : Inport
  Fus_ObjVy_Single : Inport  Port=2
  Fus_TargetState : Inport  Port=3
  Veh_YawRate : Inport  Port=4
  Fus_ObsVyStd : Inport  Port=5
  Fus_ObjDy_Single : Inport  Port=6
  Veh_AEBFunState : Inport  Port=7
  If : If
  If Action Subsystem : Subsystem  TreatAsAtomicUnit=on
  Merge : Merge
  Merge3 : Merge
  Subsystem : Subsystem
  Subsystem3 : Subsystem
  Fus_ObjVy : Outport
  Vy_CorrectFlg : Outport  Port=2
  wires:
  Fus_TargetState/1 -> Subsystem3/4(Fus_TargetState)
  Subsystem3/2(Vy_CorrectFlg) -> Merge3/2
  Merge3/1 -> Vy_CorrectFlg/1
  If Action Subsystem/2(Vy_CorrectFlg) -> Merge3/1
  Subsystem3/1(Fus_ObjVy) -> Merge/2
  Merge/1 -> Fus_ObjVy/1
  If Action Subsystem/1(Fus_ObjVy) -> Merge/1
  If/2 -> Subsystem3/Action
  If/1 -> If Action Subsystem/Action
  Veh_AEBFunState/1 -> Subsystem3/3(Veh_AEBFunState)
  Fus_ObjDy_Single/1 -> Subsystem3/2(Fus_ObjDy_Single)
  Fus_ObjVy_Single/1 -> Subsystem3/1(Fus_ObjVy_Single), If Action Subsystem/1(Fus_ObjVy_Single)
  Obj_ID/1 -> Subsystem/1(Obj_ID)
  Subsystem/1(Obj_Invalid) -> If/1
  Fus_ObsVyStd/1 -> Subsystem3/6(Fus_ObsVyStd)
  Veh_YawRate/1 -> Subsystem3/5(Veh_YawRate)

== system TosAeb/AEB_Objsel/TA_Processor/PreProcessor/ObjectPrecessor/TAObjInfoCalc/For Each Subsystem/Info_Check1/Basic_Info_check/AbsSpd ==
  ObsVelXAbs_s : Inport
  ObsVelYAbs_s : Inport  Port=2
  Vhe_Spd : Inport  Port=3
  Obj_Id : Inport  Port=4
  Veh_YawRate : Inport  Port=5
  Obj_VxCr : Inport  Port=6
  Obj_VyCr : Inport  Port=7
  AbsVx_correct : Subsystem
  AbsVy_correct : Subsystem
  Terminator : Terminator
  Fus_ObjVxAbs : Outport
  Fus_ObjVyAbs : Outport  Port=2
  wires:
  ObsVelXAbs_s/1 -> AbsVx_correct/1(ObsVelXAbs_s)
  Vhe_Spd/1 -> AbsVx_correct/2(Vhe_Spd)
  Obj_VxCr/1 -> AbsVx_correct/3(Obj_VxCr)
  ObsVelYAbs_s/1 -> AbsVy_correct/1(ObsVelYAbs_s)
  Veh_YawRate/1 -> AbsVy_correct/2(Veh_YawRate)
  Obj_VyCr/1 -> AbsVy_correct/3(Obj_VyCr)
  AbsVx_correct/1(Fus_ObjVxAbs) -> Fus_ObjVxAbs/1
  AbsVy_correct/1(Fus_ObjVyAbs) -> Fus_ObjVyAbs/1
  Obj_Id/1 -> Terminator/1

== system TosAeb/AEB_Objsel/TA_Processor/PreProcessor/ObjectPrecessor/TAObjInfoCalc/For Each Subsystem/Info_Check1/Basic_Info_check/AccCorrect ==
  ObsAccX_s : Inport
  ObsAccY_s : Inport  Port=2
  Veh_AccX : Inport  Port=3
  Veh_AccY : Inport  Port=4
  Obj_vy : Inport  Port=5
  Obj_Vx : Inport  Port=6
  Veh_AEBSta : Inport  Port=7
  Obj_Id : Inport  Port=8
  Acc_XY : Subsystem
  Fus_ObjAy : Outport
  Fus_ObjAx : Outport  Port=2
  wires:
  ObsAccY_s/1 -> Acc_XY/1(ObsAccY_s)
  Obj_vy/1 -> Acc_XY/2(Obj_vy)
  Veh_AEBSta/1 -> Acc_XY/7(Veh_AEBSta)
  ObsAccX_s/1 -> Acc_XY/3(ObsAccX_s)
  Veh_AccX/1 -> Acc_XY/5(Veh_AccX)
  Obj_Vx/1 -> Acc_XY/4(Obj_Vx)
  Veh_AccY/1 -> Acc_XY/6(Veh_AccY)
  Acc_XY/1(Fus_ObjAy) -> Fus_ObjAy/1
  Acc_XY/2(Fus_ObjAx) -> Fus_ObjAx/1
  Obj_Id/1 -> Acc_XY/8(Obj_Id)

== system TosAeb/AEB_Objsel/TA_Processor/PreProcessor/ObjectPrecessor/TAObjInfoCalc/For Each Subsystem/Info_Check1/Basic_Info_check/DxCorrect ==
  Obj_ID : Inport
  Fus_ObjDx_Single : Inport  Port=2
  Fus_ObjDxQua_Single : Inport  Port=3
  Fus_ObjVx_Single : Inport  Port=4
  Veh_AEBFunState : Inport  Port=5
  Obj_AccX : Inport  Port=6
  If : If
  Merge : Merge
  Merge3 : Merge
  Subsystem : Subsystem
  Terminator : Terminator
  dx_charge : Subsystem
  dx_nocharge : Subsystem  TreatAsAtomicUnit=on
  Fus_ObjDx : Outport
  Dx_CorrectFlg : Outport  Port=2
  wires:
  Merge3/1 -> Dx_CorrectFlg/1
  dx_charge/2(Dx_CorrectFlg) -> Merge3/2
  dx_nocharge/2(Dx_CorrectFlg) -> Merge3/1
  dx_charge/1(Fus_ObjDx) -> Merge/2
  dx_nocharge/1(Fus_ObjDx) -> Merge/1
  If/2 -> dx_charge/Action
  If/1 -> dx_nocharge/Action
  Veh_AEBFunState/1 -> dx_charge/4(Veh_AEBFunState)
  Fus_ObjVx_Single/1 -> dx_charge/3(Fus_ObjVx_Single)
  Fus_ObjDxQua_Single/1 -> dx_charge/2(Fus_ObjDxStd)
  Fus_ObjDx_Single/1 -> dx_nocharge/1(Fus_ObjDx_Single), dx_charge/1(Fus_ObjDx_Single)
  Obj_ID/1 -> Subsystem/1(Obj_ID)
  Subsystem/1(Obj_Invalid) -> If/1
  Merge/1 -> Fus_ObjDx/1
  Obj_AccX/1 -> Terminator/1

== system TosAeb/AEB_Objsel/TA_Processor/PreProcessor/ObjectPrecessor/TAObjInfoCalc/For Each Subsystem/Info_Check1/Basic_Info_check/DxCorrect1 ==
  Obj_ID : Inport
  Fus_ObjDx_Single : Inport  Port=2
  Fus_ObjDxQua_Single : Inport  Port=3
  Fus_ObjVx_Single : Inport  Port=4
  Veh_AEBFunState : Inport  Port=5
  Obj_AccX : Inport  Port=6
  Compare To Constant : simulink/Logic and Bit Operations/Compare To Constant  relop=~=  const=0  OutDataTypeStr=boolean
  If : If
  MATLAB Function1 : MATLAB Function  TreatAsAtomicUnit=on
  Merge : Merge
  Merge3 : Merge
  Relational Operator : RelationalOperator  Operator=~=  OutDataTypeStr=boolean  RndMeth=Simplest
  Subsystem : Subsystem
  Terminator : Terminator
  Terminator1 : Terminator
  Unit Delay : UnitDelay  SampleTime=-1  HasFrameUpgradeWarning=on
  dx_charge : Subsystem
  dx_nocharge : Subsystem  TreatAsAtomicUnit=on
  Fus_ObjDx : Outport
  Dx_CorrectFlg : Outport  Port=2
  wires:
  Merge/1 -> Terminator/1
  MATLAB Function1/2(RR_filtered) -> Terminator1/1  "RangeRate"
  MATLAB Function1/1(R_filtered) -> Fus_ObjDx/1  "RangeFiltered"
  Compare To Constant/1 -> MATLAB Function1/5(targetPresent)
  Relational Operator/1 -> MATLAB Function1/4(targetChanged)
  Unit Delay/1 -> Relational Operator/2
  Obj_AccX/1 -> MATLAB Function1/3(rangeAccel)
  Subsystem/1(Obj_Invalid) -> If/1
  Obj_ID/1 -> Subsystem/1(Obj_ID), Relational Operator/1, Compare To Constant/1, Unit Delay/1
  Fus_ObjDx_Single/1 -> dx_charge/1(Fus_ObjDx_Single), MATLAB Function1/1(range), dx_nocharge/1(Fus_ObjDx_Single)
  Fus_ObjDxQua_Single/1 -> dx_charge/2(Fus_ObjDxStd)
  Fus_ObjVx_Single/1 -> MATLAB Function1/2(rangeRate), dx_charge/3(Fus_ObjVx_Single)
  Veh_AEBFunState/1 -> dx_charge/4(Veh_AEBFunState)
  If/1 -> dx_nocharge/Action
  If/2 -> dx_charge/Action
  dx_nocharge/1(Fus_ObjDx) -> Merge/1
  dx_charge/1(Fus_ObjDx) -> Merge/2
  dx_nocharge/2(Dx_CorrectFlg) -> Merge3/1
  dx_charge/2(Dx_CorrectFlg) -> Merge3/2
  Merge3/1 -> Dx_CorrectFlg/1

== system TosAeb/AEB_Objsel/TA_Processor/PreProcessor/ObjectPrecessor/TAObjInfoCalc/For Each Subsystem/Info_Check1/Basic_Info_check/DyCorrect ==
  Obj_ID : Inport
  Fus_ObjDy_Single : Inport  Port=2
  Fus_ObsDyStd : Inport  Port=3
  Veh_AEBFunState : Inport  Port=4
  Fus_ObjVy_Single : Inport  Port=5
  If : If
  Merge : Merge
  Merge3 : Merge
  Subsystem : Subsystem
  dy_charge : Subsystem
  dy_nocharge : Subsystem  TreatAsAtomicUnit=on
  Fus_ObjDy : Outport
  Dy_CorrectFlg : Outport  Port=2
  wires:
  Merge3/1 -> Dy_CorrectFlg/1
  dy_charge/2(Dy_CorrectFlg) -> Merge3/2
  dy_nocharge/2(Dy_CorrectFlg) -> Merge3/1
  dy_charge/1(Fus_ObjDy) -> Merge/2
  Merge/1 -> Fus_ObjDy/1
  dy_nocharge/1(Fus_ObjDy) -> Merge/1
  Veh_AEBFunState/1 -> dy_charge/4(Veh_AEBFunState)
  Fus_ObjVy_Single/1 -> dy_charge/3(Fus_ObjVy_Single)
  Fus_ObsDyStd/1 -> dy_charge/2(Fus_ObsDyStd)
  Fus_ObjDy_Single/1 -> dy_charge/1(Fus_ObjDy_Single), dy_nocharge/1(Fus_ObjDy_Single)
  If/2 -> dy_charge/Action
  If/1 -> dy_nocharge/Action
  Subsystem/1(Obj_Invalid) -> If/1
  Obj_ID/1 -> Subsystem/1(Obj_ID)

== system TosAeb/AEB_Objsel/TA_Processor/PreProcessor/ObjectPrecessor/TAObjInfoCalc/For Each Subsystem/Info_Check1/Basic_Info_check/HeadAngle ==
  Obj_ID : Inport
  Fus_Type : Inport  Port=2
  ObsAngle_s : Inport  Port=3
  ObsAngleSTD_s : Inport  Port=4
  Obj_VxCr : Inport  Port=5
  Obj_VyCr : Inport  Port=6
  If Action Subsystem : Subsystem  TreatAsAtomicUnit=on
  If Action Subsystem1 : Subsystem  TreatAsAtomicUnit=on
  If1 : If
  Merge : Merge
  Subsystem : Subsystem
  Fus_ObjHeadAngle : Outport
  wires:
  Subsystem/1(Obj_Invalid) -> If1/1
  Obj_ID/1 -> Subsystem/1(Obj_ID)
  If1/1 -> If Action Subsystem/Action
  If1/2 -> If Action Subsystem1/Action
  Obj_VxCr/1 -> If Action Subsystem1/4(Obj_VxCr)
  Obj_VyCr/1 -> If Action Subsystem1/5(Obj_VyCr)
  ObsAngleSTD_s/1 -> If Action Subsystem1/3(ObsAngleSTD_s)
  ObsAngle_s/1 -> If Action Subsystem1/2(ObsAngle_s)
  Fus_Type/1 -> If Action Subsystem1/1(Fus_Type)
  If Action Subsystem/1(Fus_ObjHeadAngle) -> Merge/1
  If Action Subsystem1/1(Fus_ObjHeadAngle) -> Merge/2
  Merge/1 -> Fus_ObjHeadAngle/1
